simulink model "ofdm_txrx_mimo_2x"  (Simulink 6.6)
settings: Solver=VariableStepDiscrete  StartTime=0.0  StopTime=20e3  RelTol=1e-3
PreLoadFcn:
  | ofdm_rx_mimo_init
InitFcn:
  | ofdm_rx_mimo_init
StartFcn:
  | ofdm_rx_mimo_init
values from neighbouring files (symbols this model uses):
  BER_RAM_init_size = 2048    [ofdm_tx_mimo_init.m]
  CPLength = 16    [ofdm_tx_mimo_init.m]
  CRCPolynomial = hex2dec('04c11db7')    [ofdm_tx_mimo_init.m]
  freqOffset_track_filtCoef_i = hex2dec('2500')/2^32    [ofdm_rx_mimo_init.m]
  freqOffset_track_filtCoef_p = hex2dec('d0000')/2^32    [ofdm_rx_mimo_init.m]
  hi = zeros(1,length(cci))    [ofdm_rx_mimo_init.m]
  hr = zeros(1,length(ccr))    [ofdm_rx_mimo_init.m]
  max_CP_length = 16    [ofdm_tx_mimo_init.m]
  max_num_baseRateSymbols = 31    [ofdm_tx_mimo_init.m]
  max_num_subcarriers = 64    [ofdm_tx_mimo_init.m]
  max_num_trainingSymbols = 15    [ofdm_tx_mimo_init.m]
  max_numBytes = 16384    [ofdm_tx_mimo_init.m]
  max_OFDM_symbols = 2047    [ofdm_tx_mimo_init.m]
  modConstellation_qam16 = .75*[1 3 -1 -3]./3    [ofdm_tx_mimo_init.m]
  modConstellation_qam256 = 0.9375*[3 1 5 7 11 9 13 15 -3 -1 -5 -7 -11 -9 -13 -15]./15    [ofdm_tx_mimo_init.m]
  modConstellation_qam64 = 0.875*[3 1 5 7 -3 -1 -5 -7]./7    [ofdm_tx_mimo_init.m]
  modConstellation_qpsk = [1 -1]./sqrt(2)    [ofdm_tx_mimo_init.m]
  numBaseRateSymbols = 2    [ofdm_tx_mimo_init.m]
  numSubcarriers = 64    [ofdm_tx_mimo_init.m]
  numTrainingSymbols = 2    [ofdm_tx_mimo_init.m]
  pilot1_indicies = 7 + ( (64-7) * 2^16)    (= 3735559)    [ofdm_tx_mimo_init.m]
  pilot2_indicies = 21 + ( (64-21) * 2^16)    (= 2818069)    [ofdm_tx_mimo_init.m]
  pilotValue_neg = hex2dec('8000') + (2^16 * 0)    [ofdm_tx_mimo_init.m]
  pilotValue_pos = hex2dec('7FFF') + (2^16 * 0)    [ofdm_tx_mimo_init.m]
  pkt_crossCorr_thresh = 0.7    [ofdm_rx_mimo_init.m]
  pkt_energy_thresh = 0    [ofdm_rx_mimo_init.m]
  pkt_numPayloadBytes = 1400+42-6    (= 1436)    [ofdm_tx_mimo_init.m]
  pktByteNums = numHeaderBytes +  				byteIndex_numPayloadBytes(1)*2^8 +  				byteIndex_numPayloadBytes(2)*2^16 +  				byteIndex_simpleDynModMasks * 2^24    [ofdm_rx_mimo_init.m]
  PktDet_Delay = 26 + Symbol_Timing_Offset*2^7    (= 1050)    [ofdm_rx_mimo_init.m]
  preamble = 6*[0 shortsyms_10 longsyms_2]    [ofdm_tx_mimo_init.m]
  preamble_I = real(preamble)    [ofdm_tx_mimo_init.m]
  preamble_Q = imag(preamble)    [ofdm_tx_mimo_init.m]
  RAM_init_size = max_numBytes    (= 16384)    [ofdm_tx_mimo_init.m]
  reg_Interrupt_PktBuf_Control = reg_InterruptControl +  	1 * 2^16 +  	0 * 2^24    (= 65656)    [ofdm_rx_mimo_init.m]
  rx_controlBits = 1 * 2  		 + 1 * 4  		 + 0 * 8  		 + 1 * 16 * rx_SISO_Mode  		 + 1 * 32  		 + 1 * 64  		 + 0 * 128  		 + 1 * 256  		 + 0 * 512  		 + 1 * 1024  		 + 0 * 2048  		 + 1 * 4096  		 + 1 * 8192  		 + 0 * 16384  		 + 1 * 65536  		 + 1 * 131072  		 + 0 * 262144  		 + 1 * 524288    [ofdm_rx_mimo_init.m]
  Rx_PhaseNoiseTrack_K = 0.6094    [ofdm_rx_mimo_init.m]
  rx_postEq_scaling = round(rxScaling*2^11) * (1 + 2^16)    [ofdm_rx_mimo_init.m]
  RxDataScrambling_Seq = [40 198 78 63 82 173 102 245 48 111 172 115 147 230 216 93 72 65 62 2 205 242 122 90 128 83 105 97 73 10 5 252]    [ofdm_tx_mimo_init.m]
  simOnly_numSamples = length(preamble)+( (numSubcarriers+CPLength)*(numTrainingSymbols + numBaseRateSymbols + numFullRateSymbols/2) )    [ofdm_tx_mimo_init.m]
  train = [train train]    [ofdm_tx_mimo_init.m]
  tx_controlBits = (antB_preambleShift * 2^4) + (2^0 * tx_SISO_Mode) + 4*0 + 8*1    (= 73)    [ofdm_tx_mimo_init.m]
  TxDataScrambling_Seq = [40 198 78 63 82 173 102 245 48 111 172 115 147 230 216 93 72 65 62 2 205 242 122 90 128 83 105 97 73 10 5 252]    [ofdm_tx_mimo_init.m]
  txReg_symbolCounts = (2^16 * numFullRateSymbols) + (2^8 *numBaseRateSymbols ) + numTrainingSymbols    [ofdm_tx_mimo_init.m]
  TxRx_FFTScaling = bin2dec('010111') + (bin2dec('000101') * 2^6)    [ofdm_tx_mimo_init.m]
undefined symbols (not defined in the file or in its folder): AntA, AntB, BaseRate, CRC_table_gen, gcbh, intfilt, pktTiming_count_payloadStart, pktTiming_symbol_length, xlBlockLoadCallback, xlGetSimulinkPeriod
libraries: xbsIndex_r4 x3973, dspsigops x4, commchan3 x2, commrflib2 x2, dspsrcs4 x1
(part 9 of 38)

== system ofdm_txrx_mimo_2x/OFDM Rx MIMO/Equalizer & Packetizer/Packet_Constructor ==
  note: The index keeps track of the first unused location in the buffer, indexed from zero.
      |   I.e. if there are 5 bits in the buffer occupying 0 to 4, the index will be 5.
      | This is used to select which byte out of the 15 bits in the buffer will make it into the RAM block.
  I : Inport
  Q : Inport  Port=2
  Xk_index : Inport  Port=3
  Vin : Inport  Port=4
  Sym Index : Inport  Port=5
  Start : Inport  Port=6
  "  " : Subsystem  mask{period=1}
  >=8? : xbsIndex_r4/Slice  Orientation=up  nbits=1  boolean_output=off  mode=Lower Bit Location + Width  bit1=0  base1=MSB of Input  bit0=3  base0=LSB of Input  dbl_ovrd=off  block_type=slice
      settings: infoedit="Extracts a given range of bits from each input sample and presents it at the output.  The output type is ordinarily unsigned with binary point at zero, but can be Boolean when the slice is one bit wide.<P><P>Hardware notes: In hardware this block costs nothing."  block_version=VER_STRING_GOES_HERE
  AddSub1 : xbsIndex_r4/AddSub  mode=Subtraction  use_carryin=off  use_carryout=off  en=off  latency=0  precision=User Defined  arith_type=Unsigned  n_bits=4  bin_pt=0  quantization=Truncate  overflow=Wrap  dbl_ovrd=off  use_behavioral_HDL=on  pipelined=off  use_rpm=on  hw_selection=Fabric  block_type=addsub
      settings: xl_area=[2 0 0 4 0 0 0]  block_version=VER_STRING_GOES_HERE
  AddSub3 : xbsIndex_r4/AddSub  mode=Addition  use_carryin=off  use_carryout=off  en=off  latency=0  precision=User Defined  arith_type=Unsigned  n_bits=4  bin_pt=0  quantization=Truncate  overflow=Wrap  dbl_ovrd=off  use_behavioral_HDL=on  pipelined=off  use_rpm=on  hw_selection=Fabric  block_type=addsub
      settings: xl_area=[2 0 0 4 0 0 0]  block_version=VER_STRING_GOES_HERE
  Assert1 : xbsIndex_r4/Assert  Orientation=left  assert_type=off  type_source=Explicitly  arith_type="Signed  (2's comp)"  n_bits=16  bin_pt=14  assert_rate=on  rate_source=Explicitly  period=2  output_port=on  block_type=assert
      settings: infoedit=Asserts a user-defined sample rate and/or type on a signal.<P><P>Hardware notes: In hardware this block costs nothing.  block_version=VER_STRING_GOES_HERE
  Assert2 : xbsIndex_r4/Assert  assert_type=off  type_source=Explicitly  arith_type="Signed  (2's comp)"  n_bits=16  bin_pt=14  assert_rate=on  rate_source=Explicitly  period=2  output_port=on  block_type=assert
      settings: infoedit=Asserts a user-defined sample rate and/or type on a signal.<P><P>Hardware notes: In hardware this block costs nothing.  block_version=VER_STRING_GOES_HERE
  Barrel Shifter : Subsystem
  Convert2 : xbsIndex_r4/Convert  Orientation=down  arith_type=Boolean  n_bits=8  bin_pt=6  quantization=Truncate  overflow=Wrap  latency=0  dbl_ovrd=off  pipeline=off  block_type=convert
      settings: xl_area=[0 0 0 0 0 0 0]  block_version=VER_STRING_GOES_HERE
  Convert3 : xbsIndex_r4/Convert  Orientation=down  arith_type=Unsigned  n_bits=3  bin_pt=0  quantization=Truncate  overflow=Wrap  latency=0  dbl_ovrd=off  pipeline=off  block_type=convert
      settings: xl_area=[0 0 0 0 0 0 0]  block_version=VER_STRING_GOES_HERE
  Counter1 : xbsIndex_r4/Counter  cnt_type=Free Running  cnt_to=Inf  operation=Up  start_count=0  cnt_by_val=1  arith_type=Unsigned  n_bits=ceil(log2(RAM_init_size))  bin_pt=0  load_pin=off  rst=on  en=on  explicit_period=off  period=2  dbl_ovrd=off  use_behavioral_HDL=off  use_rpm=on  implementation=Fabric  block_type=counter
      settings: xl_area=[5 10 0 10 0 0 0]  block_version=VER_STRING_GOES_HERE
  Data Buffer : Subsystem
  Delay1 : xbsIndex_r4/Delay  en=off  latency=1  dbl_ovrd=off  reg_retiming=off  block_type=delay
      settings: infoedit=Hardware notes: A delay line is a chain, each link of which is an SRL16 followed by a flip-flop. If register retiming is enabled, the delay line is a chain of flip-flops.  xl_area=[1 1 0 0 0 0 0]  block_version=VER_STRING_GOES_HERE
  Delay2 : xbsIndex_r4/Delay  en=off  latency=1  dbl_ovrd=off  reg_retiming=off  block_type=delay
      settings: infoedit=Hardware notes: A delay line is a chain, each link of which is an SRL16 followed by a flip-flop. If register retiming is enabled, the delay line is a chain of flip-flops.  xl_area=[1 1 0 0 0 0 0]  block_version=VER_STRING_GOES_HERE
  Delay3 : xbsIndex_r4/Delay  en=off  latency=1  dbl_ovrd=off  reg_retiming=off  block_type=delay
      settings: infoedit=Hardware notes: A delay line is a chain, each link of which is an SRL16 followed by a flip-flop. If register retiming is enabled, the delay line is a chain of flip-flops.  xl_area=[1 1 0 0 0 0 0]  block_version=VER_STRING_GOES_HERE
  Hard Decision : Subsystem
  Inverter : xbsIndex_r4/Inverter  en=off  latency=0  dbl_ovrd=off  block_type=inv
      settings: xl_area=[0 0 0 0 0 0 0]  block_version=VER_STRING_GOES_HERE
  Inverter1 : xbsIndex_r4/Inverter  en=off  latency=0  dbl_ovrd=off  block_type=inv
      settings: xl_area=[0 0 0 0 0 0 0]  block_version=VER_STRING_GOES_HERE
  Logical1 : xbsIndex_r4/Logical  Orientation=down  logical_function=AND  inputs=3  en=off  latency=0  precision=Full  arith_type=Unsigned  n_bits=8  bin_pt=2  align_bp=on  dbl_ovrd=off  block_type=logical
      settings: xl_area=[1 0 0 1 0 0 0]  block_version=VER_STRING_GOES_HERE
  Logical2 : xbsIndex_r4/Logical  logical_function=AND  inputs=3  en=off  latency=0  precision=Full  arith_type=Unsigned  n_bits=8  bin_pt=2  align_bp=on  dbl_ovrd=off  block_type=logical
      settings: xl_area=[1 0 0 1 0 0 0]  block_version=VER_STRING_GOES_HERE
  Logical3 : xbsIndex_r4/Logical  logical_function=AND  inputs=2  en=off  latency=0  precision=Full  arith_type=Unsigned  n_bits=8  bin_pt=2  align_bp=on  dbl_ovrd=off  block_type=logical
      settings: xl_area=[1 0 0 1 0 0 0]  block_version=VER_STRING_GOES_HERE
  Logical4 : xbsIndex_r4/Logical  logical_function=OR  inputs=3  en=off  latency=0  precision=Full  arith_type=Unsigned  n_bits=8  bin_pt=2  align_bp=on  dbl_ovrd=off  block_type=logical
      settings: xl_area=[4 0 0 4 0 0 0]  block_version=VER_STRING_GOES_HERE
  Modulation RAM : Subsystem
  Pkt Interrupt Outputs : Subsystem
  Register : xbsIndex_r4/Register  init=0  rst=off  en=off  dbl_ovrd=off  block_type=register
      settings: block_version=8.2.02
  Relational : xbsIndex_r4/Relational  mode=a<b  en=off  latency=0  dbl_ovrd=off  block_type=relational
      settings: xl_area=[6 0 0 11 0 0 0]  block_version=VER_STRING_GOES_HERE
  Shift8 : xbsIndex_r4/Shift  Orientation=left  shift_dir=Left  shift_bits=3  en=off  latency=0  precision=User Defined  arith_type=Unsigned  n_bits=4  bin_pt=0  quantization=Truncate  overflow=Wrap  dbl_ovrd=off  block_type=shift
      settings: xl_area=[0 0 0 0 0 0 0]  block_version=VER_STRING_GOES_HERE
  Slicer : Subsystem
  index acc1 : xbsIndex_r4/Accumulator  operation=Add  n_bits=4  overflow=Wrap  scale=1  rst=on  hasbypass=off  en=on  dbl_ovrd=off  use_behavioral_HDL=on  implementation=Fabric  block_type=accum
      settings: infoedit="Adder or subtractor-based accumulator.   Output type and binary point position match the input.<P><P>Hardware notes: When \"Reinitialize with input 'b' on reset\" is selected, the accumulator is forced to run at the system rate even if the input 'b' is running at a slower rate."  xl_area=[2 4 0 4 0 0 0]  block_version=VER_STRING_GOES_HERE
  done : Outport
  wires:
  Delay2/1 -> AddSub1/1, AddSub3/1, Barrel Shifter/1(ShiftSel)
  Data Buffer/1(numBytes) -> Relational/2
  Logical4/1 -> index acc1/2, Counter1/1, Data Buffer/4(Rst)
  AddSub1/1 -> index acc1/1
  Shift8/1 -> AddSub1/2
  index acc1/1 -> AddSub3/2
  AddSub3/1 -> Assert2/1
  >=8?/1 -> Shift8/1, Convert2/1
  Barrel Shifter/1(Q) -> Slicer/2(D)
  Logical2/1 -> Data Buffer/2(Payload_WE)
  Slicer/1(Q) -> Data Buffer/3(Payload_Data)
  "  "/1(pulse) -> Register/1
  Inverter/1 -> "  "/1(signal)
  Convert2/1 -> Logical1/2, Logical2/2
  Logical1/1 -> Counter1/2
  Logical3/1 -> Logical2/1, Logical1/3, Inverter/1
  Counter1/1 -> Data Buffer/1(Payload_Addr), Relational/1
  Convert3/1 -> Slicer/1(SliceSel)
  Modulation RAM/1(mod) -> Hard Decision/1(Mod Sel), Delay2/1
  Xk_index/1 -> Modulation RAM/1(Xk_index)
  Sym Index/1 -> Modulation RAM/2(Sym Index)
  Hard Decision/1(bits) -> Barrel Shifter/2(D)
  I/1 -> Hard Decision/2(I)
  Q/1 -> Hard Decision/3(Q)
  Start/1 -> Logical4/1
  [rx_global_reset] -> Logical4/2, Logical4/3
  Data Buffer/2(CRCError) -> Pkt Interrupt Outputs/2(CRC-Err)
  Assert2/1 -> Convert3/1, >=8?/1
  Vin/1 -> Delay3/1
  Delay1/1 -> Barrel Shifter/3(en), index acc1/3, Logical1/1, Logical2/3
  Delay3/1 -> Delay1/1
  Register/1 -> done/1, Pkt Interrupt Outputs/1(PktDone)
  Data Buffer/6(modMask) -> Modulation RAM/3(FullRateMasks)
  Data Buffer/5(ResetRx) -> Assert1/1
  Relational/1 -> Logical3/1
  Inverter1/1 -> Logical3/2
  Assert1/1 -> Inverter1/1
  Data Buffer/3(GoodHeader) -> Pkt Interrupt Outputs/3(GoodHeader)
  Data Buffer/4(BadHeader) -> Pkt Interrupt Outputs/4(BadHeader)

== system ofdm_txrx_mimo_2x/OFDM Rx MIMO/Equalizer & Packetizer/Phase Adjust ==
  Phase : Inport
  I : Inport  Port=2
  Q : Inport  Port=3
  SubCar Index : Inport  Port=4
  Vin : Inport  Port=5
  Sym Index : Inport  Port=6
  ComplexMult_x1 : Subsystem
  Delay{1..4} (4 alike) : xbsIndex_r4/Delay  en=off  latency=1  dbl_ovrd=off  reg_retiming=off  block_type=delay
      settings: infoedit=Hardware notes: A delay line is a chain, each link of which is an SRL16 followed by a flip-flop. If register retiming is enabled, the delay line is a chain of flip-flops.  xl_area=[0, 0, 0, 0, 0, 0, 0]  block_version=VER_STRING_GOES_HERE
  Delay5 : xbsIndex_r4/Delay  en=off  latency=4  dbl_ovrd=off  reg_retiming=off  block_type=delay
      settings: infoedit=Hardware notes: A delay line is a chain, each link of which is an SRL16 followed by a flip-flop. If register retiming is enabled, the delay line is a chain of flip-flops.  xl_area=[0, 0, 0, 0, 0, 0, 0]  block_version=VER_STRING_GOES_HERE
  Delay6 : xbsIndex_r4/Delay  en=off  latency=4  dbl_ovrd=off  reg_retiming=off  block_type=delay
      settings: infoedit=Hardware notes: A delay line is a chain, each link of which is an SRL16 followed by a flip-flop. If register retiming is enabled, the delay line is a chain of flip-flops.  xl_area=[0, 0, 0, 0, 0, 0, 0]  block_version=VER_STRING_GOES_HERE
  Delay7 : xbsIndex_r4/Delay  en=off  latency=4  dbl_ovrd=off  reg_retiming=off  block_type=delay
      settings: infoedit=Hardware notes: A delay line is a chain, each link of which is an SRL16 followed by a flip-flop. If register retiming is enabled, the delay line is a chain of flip-flops.  xl_area=[0, 0, 0, 0, 0, 0, 0]  block_version=VER_STRING_GOES_HERE
  Phase Adjust : Scope  NumInputPorts=7
      settings: TimeRange=1800  YMin=-0.75~0.425~-1~-1.25~-1.25~-1.25~-1  YMax=0.75~0.65~1~1.25~1.25~1.25~1  SaveName=ScopeData67
  Phase Calc : Subsystem
  SineCosine : xbsIndex_r4/SineCosine  mode=Sine and Cosine  neg_sin=on  neg_cos=off  n_bits=16  sym_output=off  en=off  latency=1  dbl_ovrd=off  explicit_period=off  period=1  mem_type=Block RAM  pipeline=off  use_rpm=on  block_type=sincos
      settings: block_version=8.2.02
  done{1..2,6..10} (7 alike) : xbsIndex_r4/Gateway Out  hdl_port=off  timing_constraint=None  locs_specified=off  LOCs={}  block_type=gatewayout
      settings: infoedit="Gateway out block.  Converts Xilinx fixed point inputs into ouputs of type Simulink integer, double, or fixed point.<P><P>Hardware notes:  In hardware these blocks become top level output ports or are discarded, depending on how they are configured."  xl_area=[0 0 0 0 1 0 0]  block_version=VER_STRING_GOES_HERE
  " I" : Outport
  " Q" : Outport  Port=2
  " SubCar Index" : Outport  Port=3
  Vout : Outport  Port=4
  " Sym Index" : Outport  Port=5
  wires:
  SineCosine/2 -> done7/1, ComplexMult_x1/3(Re[B])
  Vin/1 -> Delay5/1
  Sym Index/1 -> Delay6/1
  SubCar Index/1 -> Delay7/1
  Delay7/1 -> " SubCar Index"/1
  Delay6/1 -> " Sym Index"/1
  Delay5/1 -> Vout/1
  Delay1/1 -> ComplexMult_x1/1(Re[A]), done8/1
  Delay2/1 -> ComplexMult_x1/2(Im[A]), done9/1
  SineCosine/1 -> ComplexMult_x1/4(Im[B]), done1/1
  done6/1 -> Phase Adjust/1
  done7/1 -> Phase Adjust/2
  done1/1 -> Phase Adjust/3
  done8/1 -> Phase Adjust/4
  done9/1 -> Phase Adjust/5
  done10/1 -> Phase Adjust/6
  done2/1 -> Phase Adjust/7
  ComplexMult_x1/1(Re) -> done10/1, " I"/1
  ComplexMult_x1/2(Im) -> done2/1, " Q"/1
  I/1 -> Delay4/1
  Q/1 -> Delay3/1
  Phase Calc/1(" Phase") -> SineCosine/1, done6/1
  Phase/1 -> Phase Calc/1(Phase/k)
  Delay3/1 -> Delay2/1
  Delay4/1 -> Delay1/1

== system ofdm_txrx_mimo_2x/OFDM Rx MIMO/Equalizer & Packetizer/Phase Noise Tracker ==
  note: http://ieeexplore.ieee.org/xpls/abs_all.jsp?arnumber=1683187
  note: Adapted from the phase tracking system described by Akita, Sakata and Sato
  note: http://sciencelinks.jp/j-east/article/200415/000020041504A0494348.php
  note: http://ieeexplore.ieee.org/xpls/abs_all.jsp?arnumber=1405003
  Phase Err : Inport
  Reset : Inport  Port=2
  Vin : Inport  Port=3
  Sym Index : Inport  Port=4
  Coarse Tracking : Subsystem
  Fine Tracking : Subsystem
  theta : Outport
  wires:
  Phase Err/1 -> Coarse Tracking/1(Phase Err)
  Reset/1 -> Coarse Tracking/2(Rst), Fine Tracking/4(Rst)
  Vin/1 -> Coarse Tracking/3(Vin)
  Coarse Tracking/1(phi) -> Fine Tracking/1(phi)
  Coarse Tracking/2(theta_coarse) -> Fine Tracking/2(theta_coarse)
  Sym Index/1 -> Fine Tracking/5(Sym Index)
  Coarse Tracking/3(vout) -> Fine Tracking/3(Vin)
  Fine Tracking/1(theta) -> theta/1

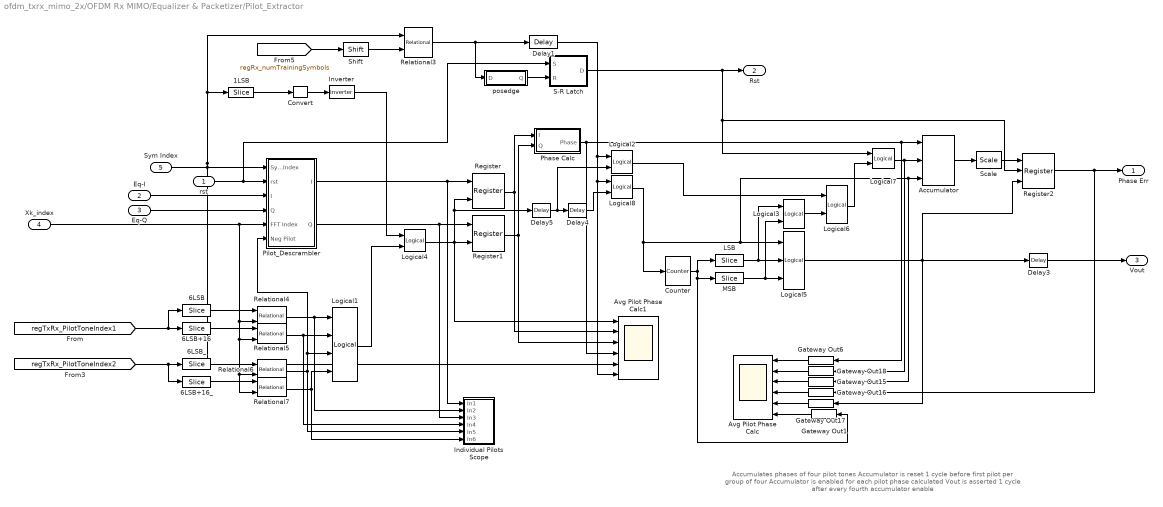
== system ofdm_txrx_mimo_2x/OFDM Rx MIMO/Equalizer & Packetizer/Pilot_Extractor ==
  note: Accumulates phases of four pilot tones
      | Accumulator is reset 1 cycle before first pilot per group of four
      | Accumulator is enabled for each pilot phase calculated
      | Vout is asserted 1 cycle after every fourth accumulator enable
  rst : Inport
  Eq-I : Inport  Port=2
  Eq-Q : Inport  Port=3
  Xk_index : Inport  Port=4
  Sym Index : Inport  Port=5
  1LSB : xbsIndex_r4/Slice  nbits=1  boolean_output=off  mode=Lower Bit Location + Width  bit1=0  base1=MSB of Input  bit0=0  base0=LSB of Input  dbl_ovrd=off  block_type=slice
      settings: infoedit="Extracts a given range of bits from each input sample and presents it at the output.  The output type is ordinarily unsigned with binary point at zero, but can be Boolean when the slice is one bit wide.<P><P>Hardware notes: In hardware this block costs nothing."  block_version=VER_STRING_GOES_HERE
  6LSB : xbsIndex_r4/Slice  nbits=ceil(log2(numSubcarriers))  boolean_output=off  mode=Lower Bit Location + Width  bit1=0  base1=MSB of Input  bit0=0  base0=LSB of Input  dbl_ovrd=off  block_type=slice
      settings: infoedit="Extracts a given range of bits from each input sample and presents it at the output.  The output type is ordinarily unsigned with binary point at zero, but can be Boolean when the slice is one bit wide.<P><P>Hardware notes: In hardware this block costs nothing."  block_version=VER_STRING_GOES_HERE
  6LSB+16 : xbsIndex_r4/Slice  nbits=ceil(log2(numSubcarriers))  boolean_output=off  mode=Lower Bit Location + Width  bit1=0  base1=MSB of Input  bit0=16  base0=LSB of Input  dbl_ovrd=off  block_type=slice
      settings: infoedit="Extracts a given range of bits from each input sample and presents it at the output.  The output type is ordinarily unsigned with binary point at zero, but can be Boolean when the slice is one bit wide.<P><P>Hardware notes: In hardware this block costs nothing."  block_version=VER_STRING_GOES_HERE
  6LSB+16_ : xbsIndex_r4/Slice  nbits=ceil(log2(numSubcarriers))  boolean_output=off  mode=Lower Bit Location + Width  bit1=0  base1=MSB of Input  bit0=16  base0=LSB of Input  dbl_ovrd=off  block_type=slice
      settings: infoedit="Extracts a given range of bits from each input sample and presents it at the output.  The output type is ordinarily unsigned with binary point at zero, but can be Boolean when the slice is one bit wide.<P><P>Hardware notes: In hardware this block costs nothing."  block_version=VER_STRING_GOES_HERE
  6LSB_ : xbsIndex_r4/Slice  nbits=ceil(log2(numSubcarriers))  boolean_output=off  mode=Lower Bit Location + Width  bit1=0  base1=MSB of Input  bit0=0  base0=LSB of Input  dbl_ovrd=off  block_type=slice
      settings: infoedit="Extracts a given range of bits from each input sample and presents it at the output.  The output type is ordinarily unsigned with binary point at zero, but can be Boolean when the slice is one bit wide.<P><P>Hardware notes: In hardware this block costs nothing."  block_version=VER_STRING_GOES_HERE
  Accumulator : xbsIndex_r4/Accumulator  operation=Add  n_bits=19  overflow=Flag as error  scale=1  rst=on  hasbypass=off  en=on  dbl_ovrd=off  use_behavioral_HDL=on  implementation=Fabric  block_type=accum
      settings: infoedit="Adder or subtractor-based accumulator.   Output type and binary point position match the input.<P><P>Hardware notes: When \"Reinitialize with input 'b' on reset\" is selected, the accumulator is forced to run at the system rate even if the input 'b' is running at a slower rate."  block_version=10.1.2
  Avg Pilot Phase Calc : Scope  NumInputPorts=6
      settings: TimeRange=8000  YMin=0~0~0~0~0~0  YMax=1.75~1~1~0.45~1~3  SaveName=ScopeData60
  Avg Pilot Phase Calc1 : Scope  NumInputPorts=6
      settings: TimeRange=8000  YMin=0~0~0~0~0~0  YMax=0.8~0.2~1~1~0.4~0.4  SaveName=ScopeData25
  Convert : xbsIndex_r4/Convert  arith_type=Boolean  n_bits=16  bin_pt=14  quantization=Truncate  overflow=Wrap  latency=0  dbl_ovrd=off  pipeline=off  block_type=convert
      settings: block_version=VER_STRING_GOES_HERE
  Counter : xbsIndex_r4/Counter  cnt_type=Free Running  cnt_to=Inf  operation=Up  start_count=0  cnt_by_val=1  arith_type=Unsigned  n_bits=2  bin_pt=0  load_pin=off  rst=off  en=on  explicit_period=off  period=1  dbl_ovrd=off  use_behavioral_HDL=off  use_rpm=off  implementation=Fabric  block_type=counter
      settings: block_version=10.1.2
  Delay1 : xbsIndex_r4/Delay  en=off  latency=7  dbl_ovrd=off  reg_retiming=off  block_type=delay
      settings: infoedit=Hardware notes: A delay line is a chain, each link of which is an SRL16 followed by a flip-flop. If register retiming is enabled, the delay line is a chain of flip-flops.  block_version=VER_STRING_GOES_HERE
  Delay3 : xbsIndex_r4/Delay  en=off  latency=1  dbl_ovrd=off  reg_retiming=off  block_type=delay
      settings: infoedit=Hardware notes: A delay line is a chain, each link of which is an SRL16 followed by a flip-flop. If register retiming is enabled, the delay line is a chain of flip-flops.  block_version=VER_STRING_GOES_HERE
  Delay4 : xbsIndex_r4/Delay  en=off  latency=1  dbl_ovrd=off  reg_retiming=off  block_type=delay
      settings: infoedit=Hardware notes: A delay line is a chain, each link of which is an SRL16 followed by a flip-flop. If register retiming is enabled, the delay line is a chain of flip-flops.  block_version=VER_STRING_GOES_HERE
  Delay5 : xbsIndex_r4/Delay  en=off  latency=8  dbl_ovrd=off  reg_retiming=off  block_type=delay
      settings: infoedit=Hardware notes: A delay line is a chain, each link of which is an SRL16 followed by a flip-flop. If register retiming is enabled, the delay line is a chain of flip-flops.  block_version=VER_STRING_GOES_HERE
  Gateway Out{1,6,15..18} (6 alike) : xbsIndex_r4/Gateway Out  Orientation=left  hdl_port=off  timing_constraint=None  locs_specified=off  LOCs={}  block_type=gatewayout
      settings: infoedit="Gateway out block.  Converts Xilinx fixed point inputs into ouputs of type Simulink integer, double, or fixed point.<P><P>Hardware notes:  In hardware these blocks become top level output ports or are discarded, depending on how they are configured."  block_version=VER_STRING_GOES_HERE
  Individual Pilots Scope : Subsystem
  Inverter : xbsIndex_r4/Inverter  en=off  latency=0  dbl_ovrd=off  block_type=inv
      settings: block_version=VER_STRING_GOES_HERE
  LSB : xbsIndex_r4/Slice  nbits=1  boolean_output=on  mode=Lower Bit Location + Width  bit1=0  base1=MSB of Input  bit0=0  base0=LSB of Input  dbl_ovrd=off  block_type=slice
      settings: infoedit="Extracts a given range of bits from each input sample and presents it at the output.  The output type is ordinarily unsigned with binary point at zero, but can be Boolean when the slice is one bit wide.<P><P>Hardware notes: In hardware this block costs nothing."  block_version=VER_STRING_GOES_HERE
  Logical1 : xbsIndex_r4/Logical  logical_function=OR  inputs=4  en=off  latency=0  precision=Full  arith_type=Unsigned  n_bits=8  bin_pt=2  align_bp=on  dbl_ovrd=off  block_type=logical
      settings: xl_area=[2 0 0 2 0 0 0]  block_version=VER_STRING_GOES_HERE
  Logical{2,4,6,8} (4 alike) : xbsIndex_r4/Logical  logical_function=AND  inputs=2  en=off  latency=0  precision=Full  arith_type=Unsigned  n_bits=8  bin_pt=2  align_bp=on  dbl_ovrd=off  block_type=logical
      settings: xl_area=[2 0 0 2 0 0 0]  block_version=VER_STRING_GOES_HERE
  Logical3 : xbsIndex_r4/Logical  logical_function=NOR  inputs=2  en=off  latency=0  precision=Full  arith_type=Unsigned  n_bits=8  bin_pt=2  align_bp=on  dbl_ovrd=off  block_type=logical
      settings: xl_area=[2 0 0 2 0 0 0]  block_version=VER_STRING_GOES_HERE
  Logical5 : xbsIndex_r4/Logical  logical_function=AND  inputs=3  en=off  latency=1  precision=Full  arith_type=Unsigned  n_bits=8  bin_pt=2  align_bp=on  dbl_ovrd=off  block_type=logical
      settings: xl_area=[2 0 0 2 0 0 0]  block_version=VER_STRING_GOES_HERE
  Logical7 : xbsIndex_r4/Logical  logical_function=OR  inputs=2  en=off  latency=0  precision=Full  arith_type=Unsigned  n_bits=8  bin_pt=2  align_bp=on  dbl_ovrd=off  block_type=logical
      settings: xl_area=[2 0 0 2 0 0 0]  block_version=VER_STRING_GOES_HERE
  MSB : xbsIndex_r4/Slice  nbits=1  boolean_output=on  mode=Upper Bit Location + Width  bit1=0  base1=MSB of Input  bit0=0  base0=LSB of Input  dbl_ovrd=off  block_type=slice
      settings: infoedit="Extracts a given range of bits from each input sample and presents it at the output.  The output type is ordinarily unsigned with binary point at zero, but can be Boolean when the slice is one bit wide.<P><P>Hardware notes: In hardware this block costs nothing."  block_version=VER_STRING_GOES_HERE
  Phase Calc : Subsystem
  Pilot_Descrambler : Subsystem
  Register : xbsIndex_r4/Register  init=0  rst=off  en=on  dbl_ovrd=off  block_type=register
      settings: block_version=VER_STRING_GOES_HERE
  Register1 : xbsIndex_r4/Register  init=0  rst=off  en=on  dbl_ovrd=off  block_type=register
      settings: block_version=VER_STRING_GOES_HERE
  Register2 : xbsIndex_r4/Register  init=0  rst=on  en=on  dbl_ovrd=off  block_type=register
      settings: block_version=VER_STRING_GOES_HERE
  Relational3 : xbsIndex_r4/Relational  mode=a>=b  en=off  latency=0  dbl_ovrd=off  block_type=relational
      settings: xl_area=[6 0 0 11 0 0 0]  block_version=VER_STRING_GOES_HERE
  Relational{4..7} (4 alike) : xbsIndex_r4/Relational  mode=a=b  en=off  latency=0  dbl_ovrd=off  block_type=relational
      settings: xl_area=[2 0 0 3 0 0 0]  block_version=VER_STRING_GOES_HERE
  S-R Latch : Subsystem
  Scale : xbsIndex_r4/Scale  scale_factor=-2  dbl_ovrd=off  block_type=scale
      settings: infoedit=Scales input by a power of two by adjusting the binary point position.<P><P>Hardware notes: In hardware this block costs nothing.  block_version=10.1.2
  Shift : xbsIndex_r4/Shift  shift_dir=Left  shift_bits=1  en=off  latency=0  precision=User Defined  arith_type=Unsigned  n_bits=1+ceil(log2(max_num_trainingSymbols))  bin_pt=0  quantization=Truncate  overflow=Flag as error  dbl_ovrd=off  block_type=shift
      settings: block_version=VER_STRING_GOES_HERE
  posedge : Subsystem
  Phase Err : Outport
  Rst : Outport  Port=2
  Vout : Outport  Port=3
  wires:
  [regTxRx_PilotToneIndex1] -> 6LSB+16/1, 6LSB/1
  [regTxRx_PilotToneIndex2] -> 6LSB_/1, 6LSB+16_/1
  6LSB_/1 -> Relational6/1
  6LSB+16_/1 -> Relational7/1
  6LSB+16/1 -> Relational5/1
  6LSB/1 -> Relational4/1
  Xk_index/1 -> Pilot_Descrambler/5(FFT Index), Relational5/2, Relational7/2, Relational6/2, Relational4/2
  Relational4/1 -> Logical1/1, Individual Pilots Scope/2(In2)
  Relational5/1 -> Logical1/2, Individual Pilots Scope/4(In4)
  Relational6/1 -> Logical1/3, Pilot_Descrambler/6(Neg Pilot), Individual Pilots Scope/5(In5)
  Relational7/1 -> Logical1/4, Individual Pilots Scope/6(In6)
  Eq-I/1 -> Pilot_Descrambler/3("I ")
  Eq-Q/1 -> Pilot_Descrambler/4("Q ")
  rst/1 -> Pilot_Descrambler/2(rst), S-R Latch/1(S)
  Pilot_Descrambler/2(Q) -> Register1/1, Individual Pilots Scope/3(In3)
  Pilot_Descrambler/1(I) -> Register/1, Individual Pilots Scope/1(In1)
  Register/1 -> Phase Calc/1(I), Avg Pilot Phase Calc1/2
  Register1/1 -> Phase Calc/2(Q), Avg Pilot Phase Calc1/3
  Sym Index/1 -> Pilot_Descrambler/1(Sym Index), Relational3/1, 1LSB/1, Avg Pilot Phase Calc1/5
  Phase Calc/1(Phase) -> Accumulator/1, Gateway Out6/1, Avg Pilot Phase Calc1/4
  Shift/1 -> Relational3/2
  [regRx_numTrainingSymbols] -> Shift/1
  Relational3/1 -> posedge/1(D), Delay1/1
  1LSB/1 -> Convert/1
  Convert/1 -> Inverter/1
  Logical4/1 -> Register1/2, Register/2, Delay5/1, Avg Pilot Phase Calc1/1
  Logical1/1 -> Logical4/2
  S-R Latch/1(D) -> Rst/1, Logical7/1, Register2/2
  posedge/1(Q) -> S-R Latch/2(R)
  Inverter/1 -> Logical4/1
  Counter/1 -> LSB/1, MSB/1, Gateway Out1/1
  LSB/1 -> Logical5/2, Logical3/1
  MSB/1 -> Logical5/3, Logical3/2
  Accumulator/1 -> Scale/1
  Gateway Out6/1 -> Avg Pilot Phase Calc/1
  Gateway Out15/1 -> Avg Pilot Phase Calc/3
  Gateway Out16/1 -> Avg Pilot Phase Calc/4
  Gateway Out17/1 -> Avg Pilot Phase Calc/5
  Gateway Out18/1 -> Avg Pilot Phase Calc/2
  Logical3/1 -> Logical6/2
  Logical7/1 -> Accumulator/2, Gateway Out18/1
  Logical6/1 -> Logical7/2
  Delay1/1 -> Logical2/1, Logical8/1, Avg Pilot Phase Calc1/6
  Scale/1 -> Register2/1
  Delay5/1 -> Delay4/1, Logical2/2
  Delay4/1 -> Logical8/2
  Logical8/1 -> Logical5/1, Accumulator/3, Gateway Out15/1, Counter/1
  Logical2/1 -> Logical6/1
  Logical5/1 -> Gateway Out17/1, Delay3/1, Register2/3
  Gateway Out1/1 -> Avg Pilot Phase Calc/6
  Register2/1 -> Phase Err/1, Gateway Out16/1
  Delay3/1 -> Vout/1

== system ofdm_txrx_mimo_2x/OFDM Rx MIMO/Equalizer & Packetizer/Scaling ==
  note: This block scales the equalized constellation so it can be easily sliced/demapped for hard decisions,
  Eq-I : Inport
  Eq-Q : Inport  Port=2
  Xk_index : Inport  Port=3
  Vin : Inport  Port=4
  Sym Index : Inport  Port=5
  " To_UFix16_11" : xbsIndex_r4/Reinterpret  force_arith_type=on  arith_type=Unsigned  force_bin_pt=on  bin_pt=11  block_type=cast
      settings: infoedit="Changes type of samples without altering their binary representation.<P><P>Hardware notes: In hardware this block costs nothing.<P><P>Example:  Suppose the input is 6 bits wide, signed, with 2 fractional bits, and the output is forced to unsigned with 0 fractional bits.  Then an input of -2.0 (1110.00 in binary 2's complement) becomes an output of 56 (111000 in binary)."  block_version=VER_STRING_GOES_HERE
  16LSB : xbsIndex_r4/Slice  nbits=16  boolean_output=off  mode=Lower Bit Location + Width  bit1=0  base1=LSB of Input  bit0=0  base0=LSB of Input  dbl_ovrd=off  block_type=slice
      settings: infoedit="Extracts a given range of bits from each input sample and presents it at the output.  The output type is ordinarily unsigned with binary point at zero, but can be Boolean when the slice is one bit wide.<P><P>Hardware notes: In hardware this block costs nothing."  block_version=VER_STRING_GOES_HERE
  16MSB : xbsIndex_r4/Slice  nbits=16  boolean_output=off  mode=Lower Bit Location + Width  bit1=0  base1=LSB of Input  bit0=16  base0=LSB of Input  dbl_ovrd=off  block_type=slice
      settings: infoedit="Extracts a given range of bits from each input sample and presents it at the output.  The output type is ordinarily unsigned with binary point at zero, but can be Boolean when the slice is one bit wide.<P><P>Hardware notes: In hardware this block costs nothing."  block_version=VER_STRING_GOES_HERE
  1LSB : xbsIndex_r4/Slice  nbits=1  boolean_output=off  mode=Lower Bit Location + Width  bit1=0  base1=MSB of Input  bit0=0  base0=LSB of Input  dbl_ovrd=off  block_type=slice
      settings: infoedit="Extracts a given range of bits from each input sample and presents it at the output.  The output type is ordinarily unsigned with binary point at zero, but can be Boolean when the slice is one bit wide.<P><P>Hardware notes: In hardware this block costs nothing."  block_version=VER_STRING_GOES_HERE
  Delay1 : xbsIndex_r4/Delay  en=off  latency=1  dbl_ovrd=off  reg_retiming=off  block_type=delay
      settings: infoedit=Hardware notes: A delay line is a chain, each link of which is an SRL16 followed by a flip-flop. If register retiming is enabled, the delay line is a chain of flip-flops.  xl_area=[0, 0, 0, 0, 0, 0, 0]  block_version=VER_STRING_GOES_HERE
  Delay3 : xbsIndex_r4/Delay  en=off  latency=1  dbl_ovrd=off  reg_retiming=off  block_type=delay
      settings: infoedit=Hardware notes: A delay line is a chain, each link of which is an SRL16 followed by a flip-flop. If register retiming is enabled, the delay line is a chain of flip-flops.  xl_area=[0, 0, 0, 0, 0, 0, 0]  block_version=VER_STRING_GOES_HERE
  Delay4 : xbsIndex_r4/Delay  en=off  latency=1  dbl_ovrd=off  reg_retiming=off  block_type=delay
      settings: infoedit=Hardware notes: A delay line is a chain, each link of which is an SRL16 followed by a flip-flop. If register retiming is enabled, the delay line is a chain of flip-flops.  xl_area=[0, 0, 0, 0, 0, 0, 0]  block_version=VER_STRING_GOES_HERE
  Down Sample1 : xbsIndex_r4/Down Sample  sample_ratio=2  sample_phase="Last Value of Frame  (most efficient)"  en=off  latency=1  dbl_ovrd=off  block_type=dsamp
      settings: block_version=VER_STRING_GOES_HERE
  Mult : xbsIndex_r4/Mult  precision=User Defined  arith_type="Signed  (2's comp)"  n_bits=symbol_unmap_nb  bin_pt=symbol_unmap_bp  quantization="Round  (unbiased: +/- Inf)"  overflow=Saturate  en=off  latency=1  dbl_ovrd=off  use_behavioral_HDL=off  use_embedded=on  opt=Speed  optimum_pipeline=off  pipeline=on  use_rpm=off  placement_style=Rectangular shape  block_type=mult
      settings: infoedit=Hardware notes: To use the internal pipeline stage of the dedicated multiplier you must select 'Pipeline for maximum performance'.  xl_area=[12 0 0 20 0 1 0]  block_version=VER_STRING_GOES_HERE
  Mult1 : xbsIndex_r4/Mult  precision=User Defined  arith_type="Signed  (2's comp)"  n_bits=symbol_unmap_nb  bin_pt=symbol_unmap_bp  quantization="Round  (unbiased: +/- Inf)"  overflow=Saturate  en=off  latency=1  dbl_ovrd=off  use_behavioral_HDL=off  use_embedded=on  opt=Speed  optimum_pipeline=off  pipeline=on  use_rpm=off  placement_style=Rectangular shape  block_type=mult
      settings: infoedit=Hardware notes: To use the internal pipeline stage of the dedicated multiplier you must select 'Pipeline for maximum performance'.  xl_area=[12 0 0 20 0 1 0]  block_version=VER_STRING_GOES_HERE
  Mux : xbsIndex_r4/Mux  inputs=2  en=off  latency=0  precision=Full  arith_type=Unsigned  n_bits=16  bin_pt=14  quantization=Truncate  overflow=Wrap  dbl_ovrd=off  block_type=mux
      settings: block_version=VER_STRING_GOES_HERE
  Scaled Constellation : Scope  NumInputPorts=4
      settings: TimeRange=1800  YMin=-1~-1~0.5~0  YMax=1~1~7.5~1  SaveName=ScopeData56
  To_UFix16_11 : xbsIndex_r4/Reinterpret  force_arith_type=on  arith_type=Unsigned  force_bin_pt=on  bin_pt=11  block_type=cast
      settings: infoedit="Changes type of samples without altering their binary representation.<P><P>Hardware notes: In hardware this block costs nothing.<P><P>Example:  Suppose the input is 6 bits wide, signed, with 2 fractional bits, and the output is forced to unsigned with 0 fractional bits.  Then an input of -2.0 (1110.00 in binary 2's complement) becomes an output of 56 (111000 in binary)."  block_version=VER_STRING_GOES_HERE
  done{2..5} (4 alike) : xbsIndex_r4/Gateway Out  hdl_port=off  timing_constraint=None  locs_specified=off  LOCs={}  block_type=gatewayout
      settings: infoedit="Gateway out block.  Converts Xilinx fixed point inputs into ouputs of type Simulink integer, double, or fixed point.<P><P>Hardware notes:  In hardware these blocks become top level output ports or are discarded, depending on how they are configured."  xl_area=[0 0 0 0 1 0 0]  block_version=VER_STRING_GOES_HERE
  I : Outport
  Q : Outport  Port=2
  " Xk_index" : Outport  Port=3
  Vout : Outport  Port=4
  " Sym Index" : Outport  Port=5
  tag scope: EQ_I=global, EQ_Q=global
  wires:
  Eq-Q/1 -> Mult1/2
  Eq-I/1 -> Mult/2
  Mult1/1 -> Q/1, [EQ_Q], done4/1
  Mult/1 -> I/1, [EQ_I], done3/1
  Mux/1 -> Mult1/1, Mult/1, done2/1
  1LSB/1 -> Mux/1, done5/1
  " To_UFix16_11"/1 -> Mux/3
  To_UFix16_11/1 -> Mux/2
  Xk_index/1 -> Delay1/1
  Delay1/1 -> " Xk_index"/1
  Vin/1 -> Delay3/1
  Delay3/1 -> Vout/1
  Sym Index/1 -> Delay4/1, 1LSB/1
  Delay4/1 -> " Sym Index"/1
  [RxReg_PostEQScaling] -> Down Sample1/1
  16LSB/1 -> To_UFix16_11/1
  16MSB/1 -> " To_UFix16_11"/1
  Down Sample1/1 -> 16LSB/1, 16MSB/1
  done3/1 -> Scaled Constellation/1
  done4/1 -> Scaled Constellation/2
  done2/1 -> Scaled Constellation/3
  done5/1 -> Scaled Constellation/4

== system ofdm_txrx_mimo_2x/OFDM Rx MIMO/Equalizer & Packetizer/posedge ==
  note: Rising Edge Detection
  D : Inport
  Delay : xbsIndex_r4/Delay  en=off  latency=1  dbl_ovrd=off  reg_retiming=off  block_type=delay
      settings: infoedit=Hardware notes: A delay line is a chain, each link of which is an SRL16 followed by a flip-flop. If register retiming is enabled, the delay line is a chain of flip-flops.  xl_area=[1 1 0 0 0 0 0]  block_version=VER_STRING_GOES_HERE
  Inverter : xbsIndex_r4/Inverter  en=off  latency=0  dbl_ovrd=off  block_type=inv
      settings: xl_area=[0 0 0 0 0 0 0]  block_version=VER_STRING_GOES_HERE
  Logical1 : xbsIndex_r4/Logical  logical_function=AND  inputs=2  en=off  latency=0  precision=Full  arith_type=Unsigned  n_bits=8  bin_pt=2  align_bp=on  dbl_ovrd=off  block_type=logical
      settings: xl_area=[1 0 0 1 0 0 0]  block_version=VER_STRING_GOES_HERE
  Q : Outport
  wires:
  Delay/1 -> Inverter/1
  Inverter/1 -> Logical1/1
  Logical1/1 -> Q/1
  D/1 -> Delay/1, Logical1/2

== system ofdm_txrx_mimo_2x/OFDM Rx MIMO/FFT & Chan Est/Addr Gen1 ==
  FFT Done : Inport
  Payload : Inport  Port=2
  NotTraining : Inport  Port=3
  1MSB : xbsIndex_r4/Slice  nbits=1  boolean_output=on  mode=Upper Bit Location + Width  bit1=0  base1=MSB of Input  bit0=0  base0=MSB of Input  dbl_ovrd=off  block_type=slice
      settings: infoedit="Extracts a given range of bits from each input sample and presents it at the output.  The output type is ordinarily unsigned with binary point at zero, but can be Boolean when the slice is one bit wide.<P><P>Hardware notes: In hardware this block costs nothing."  block_version=VER_STRING_GOES_HERE
  6LSB : xbsIndex_r4/Slice  nbits=6  boolean_output=off  mode=Lower Bit Location + Width  bit1=0  base1=LSB of Input  bit0=0  base0=LSB of Input  dbl_ovrd=off  block_type=slice
      settings: infoedit="Extracts a given range of bits from each input sample and presents it at the output.  The output type is ordinarily unsigned with binary point at zero, but can be Boolean when the slice is one bit wide.<P><P>Hardware notes: In hardware this block costs nothing."  block_version=VER_STRING_GOES_HERE
  Constant1 : xbsIndex_r4/Constant  Orientation=left  arith_type=Unsigned  const=numSubcarriers*2-1  n_bits=ceil(log2(numSubcarriers*2))  bin_pt=0  explicit_period=off  period=1  equ=P=C  opselect=C  inp2=PCIN>>17  opr=+  inp1=P  carry=CIN  dbl_ovrd=off  block_type=constant
      settings: block_version=VER_STRING_GOES_HERE
  Copy_Downsample : Subsystem
  Counter : xbsIndex_r4/Counter  cnt_type=Free Running  cnt_to=127  operation=Up  start_count=0  cnt_by_val=1  arith_type=Unsigned  n_bits=ceil(log2(numSubcarriers*2))  bin_pt=0  load_pin=off  rst=on  en=on  explicit_period=off  period=2  dbl_ovrd=off  use_behavioral_HDL=off  use_rpm=on  implementation=Fabric  block_type=counter
      settings: xl_area=[6 12 0 12 0 0 0]  block_version=VER_STRING_GOES_HERE
  Delay : xbsIndex_r4/Delay  en=off  latency=1  dbl_ovrd=off  reg_retiming=off  block_type=delay
      settings: block_version=10.1.2
  FFT Output Buffers Addrs 2 : Scope  NumInputPorts=6
      settings: TimeRange=4000  YMin=0~0~-1~0~-1~0  YMax=1~1~1~1~1~80  SaveName=ScopeData74
  Inverter2 : xbsIndex_r4/Inverter  en=off  latency=0  dbl_ovrd=off  block_type=inv
      settings: block_version=VER_STRING_GOES_HERE
  Inverter3 : xbsIndex_r4/Inverter  en=off  latency=0  dbl_ovrd=off  block_type=inv
      settings: block_version=VER_STRING_GOES_HERE
  Logical1 : xbsIndex_r4/Logical  logical_function=AND  inputs=3  en=off  latency=0  precision=Full  arith_type=Unsigned  n_bits=8  bin_pt=2  align_bp=on  dbl_ovrd=off  block_type=logical
      settings: xl_area=[0, 0, 0, 0, 0, 0, 0]  block_version=VER_STRING_GOES_HERE
  Logical10 : xbsIndex_r4/Logical  logical_function=AND  inputs=2  en=off  latency=0  precision=Full  arith_type=Unsigned  n_bits=8  bin_pt=2  align_bp=on  dbl_ovrd=off  block_type=logical
      settings: xl_area=[0, 0, 0, 0, 0, 0, 0]  block_version=VER_STRING_GOES_HERE
  Logical11 : xbsIndex_r4/Logical  logical_function=AND  inputs=2  en=off  latency=0  precision=Full  arith_type=Unsigned  n_bits=8  bin_pt=2  align_bp=on  dbl_ovrd=off  block_type=logical
      settings: xl_area=[0, 0, 0, 0, 0, 0, 0]  block_version=VER_STRING_GOES_HERE
  Logical2 : xbsIndex_r4/Logical  logical_function=OR  inputs=2  en=off  latency=0  precision=Full  arith_type=Unsigned  n_bits=8  bin_pt=2  align_bp=on  dbl_ovrd=off  block_type=logical
      settings: xl_area=[4 0 0 4 0 0 0]  block_version=VER_STRING_GOES_HERE
  Logical3 : xbsIndex_r4/Logical  logical_function=OR  inputs=2  en=off  latency=0  precision=Full  arith_type=Unsigned  n_bits=8  bin_pt=2  align_bp=on  dbl_ovrd=off  block_type=logical
      settings: xl_area=[0, 0, 0, 0, 0, 0, 0]  block_version=VER_STRING_GOES_HERE
  Logical6 : xbsIndex_r4/Logical  logical_function=AND  inputs=3  en=off  latency=0  precision=Full  arith_type=Unsigned  n_bits=8  bin_pt=2  align_bp=on  dbl_ovrd=off  block_type=logical
      settings: xl_area=[0, 0, 0, 0, 0, 0, 0]  block_version=VER_STRING_GOES_HERE
  Logical7 : xbsIndex_r4/Logical  logical_function=NAND  inputs=2  en=off  latency=0  precision=Full  arith_type=Unsigned  n_bits=8  bin_pt=2  align_bp=on  dbl_ovrd=off  block_type=logical
      settings: xl_area=[1 0 0 1 0 0 0]  block_version=VER_STRING_GOES_HERE
  Oscillator : Subsystem
  Output Sym Counter : Subsystem
  Relational : xbsIndex_r4/Relational  Orientation=left  mode=a<b  en=off  latency=0  dbl_ovrd=off  block_type=relational
      settings: block_version=VER_STRING_GOES_HERE
  S-R Latch1 : Subsystem
  done{1..4,7..8} (6 alike) : xbsIndex_r4/Gateway Out  hdl_port=off  timing_constraint=None  locs_specified=off  LOCs={}  block_type=gatewayout
      settings: infoedit="Gateway out block.  Converts Xilinx fixed point inputs into ouputs of type Simulink integer, double, or fixed point.<P><P>Hardware notes:  In hardware these blocks become top level output ports or are discarded, depending on how they are configured."  xl_area=[0 0 0 0 1 0 0]  block_version=VER_STRING_GOES_HERE
  SymIndex_out : Outport
  Rd Addr : Outport  Port=2
  Vout : Outport  Port=3
  wires:
  Counter/1 -> 6LSB/1, Relational/1, 1MSB/1
  6LSB/1 -> Rd Addr/1, Output Sym Counter/4(Read Addr), done1/1
  Constant1/1 -> Relational/2
  FFT Done/1 -> Copy_Downsample/1(D), done7/1
  [regRx_SISOmode] -> Logical7/1
  1MSB/1 -> Logical7/2
  Payload/1 -> Output Sym Counter/3(Payload), Logical6/3, done4/1, Inverter2/1
  Inverter2/1 -> Oscillator/2(rst), Logical2/1
  NotTraining/1 -> Logical1/3, Output Sym Counter/2(Not Training), done3/1
  Oscillator/1(Q) -> Logical1/2
  S-R Latch1/1(D) -> Logical10/1
  Logical1/1 -> Delay/1
  Logical10/1 -> Counter/2, Logical6/2
  Relational/1 -> Logical10/2, Inverter3/1
  Inverter3/1 -> Logical11/2
  [rx_global_reset] -> Logical2/2
  Logical2/1 -> S-R Latch1/2(R), Logical3/1
  Copy_Downsample/1(Q) -> Oscillator/1(en), Logical1/1, Output Sym Counter/1(FFT Done)
  Delay/1 -> S-R Latch1/1(S), Logical11/1
  Logical6/1 -> Vout/1, done2/1
  Logical7/1 -> Logical6/1
  done3/1 -> FFT Output Buffers Addrs 2/6
  done4/1 -> FFT Output Buffers Addrs 2/5
  done1/1 -> FFT Output Buffers Addrs 2/1
  done2/1 -> FFT Output Buffers Addrs 2/2
  done8/1 -> FFT Output Buffers Addrs 2/3
  done7/1 -> FFT Output Buffers Addrs 2/4
  Output Sym Counter/1(Sym Index) -> SymIndex_out/1, done8/1
  Logical11/1 -> Logical3/2
  Logical3/1 -> Counter/1
